simulink model "transmitter_lfsr256_v10"  (R2020a)
settings: Solver=VariableStepAuto  StartTime=0.0  StopTime=3000/128e6  RelTol=1e-3
PreLoadFcn:
  | setup_transmitter
InitFcn:
  | setup_transmitter
values from neighbouring files (symbols this model uses):
  tx_data = fi([1:1:1000], 0, 8, 0)    [setup_transmitter.m]
undefined symbols (not defined in the file or in its folder): a1, a2, a3, a4, a5, a6, a7, a8, AXI4, b1, b2, b3, b4, b5, b6, b7, b8, c1, c2, c3, c4, c5, c6, c7, c8, d1, d2, d3, d4, d5, d6, d7, d8, Lite
libraries: xbsIndex_r4 x30918, dspstat3 x66, hdlsllib x2, simulink x1
(part 1 of 157)
settings Model: Creator=David Northcote  LastModifiedBy=observer
settings SFSimCC: CustomCodeUndefinedFunction=UseInterfaceOnly

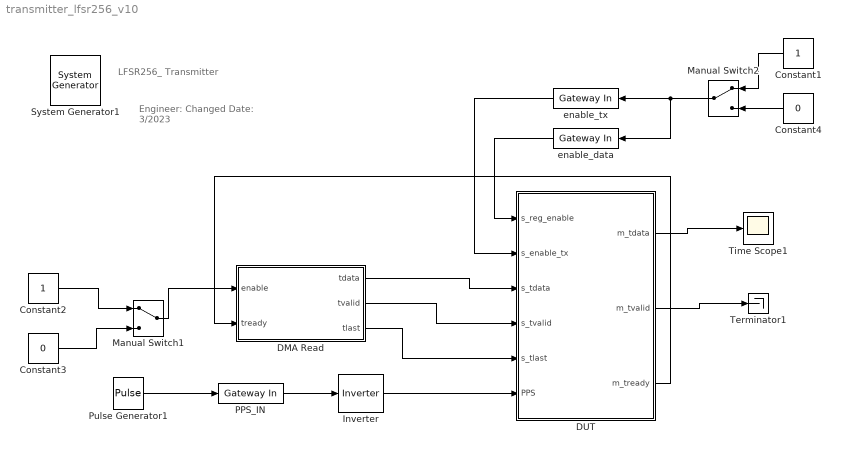
== system transmitter_lfsr256_v10 ==
  note: Engineer:	Changed
      | Date:		3/2023
  note: LFSR256_ Transmitter
  " System Generator1" : xbsIndex_r4/ System Generator  Tag=genX  xilinxfamily=zynquplusRFSOC  part=xczu48dr  speed=-2-e  package=ffvg1517  synthesis_tool=Vivado  clock_wrapper=Clock Enables  directory=/home/observer/Downloads/rfsoc4x2_projects/working_designs/rfsoc_radio_lfsr32x8_983.04MHz_PPS_G/rfsoc_radio/boards/ip/sysgen/transmitter/netlist_lfsr32x8ppsdatabuswidth8_as128  proj_type=Vivado  Synth_file=Vivado Synthesis Defaults  Impl_file=Vivado Implementation Defaults  testbench=off  simulink_period=1/128e6  sysclk_period=(1/128e6)*1e9  dcm_input_clock_period=10  incr_netlist=off  trim_vbits=Everywhere in SubSystem  dbl_ovrd=According to Block Masks  core_generation=According to Block Masks  run_coregen=off  deprecated_control=off  eval_field=0  block_type=sysgen
  Constant1 : Constant  OutDataTypeStr=boolean  SampleTime=1/(128e6)
  Constant2 : Constant  OutDataTypeStr=boolean  SampleTime=1/128e6
  Constant3 : Constant  Value=0  OutDataTypeStr=boolean  SampleTime=1/128e6
  Constant4 : Constant  Value=0  OutDataTypeStr=boolean  SampleTime=1/(128e6)
  DMA Read : Subsystem
  DUT : Subsystem
  Inverter : xbsIndex_r4/Inverter  en=off  latency=0  dbl_ovrd=off  block_type=inv
  Manual Switch1 : ManualSwitch
  Manual Switch2 : ManualSwitch
  PPS_IN : xbsIndex_r4/Gateway In  gui_display_data_type=Boolean  arith_type=Boolean  n_bits=1  bin_pt=0  preci_type=Single  exp_width=8  frac_width=24  rows=1  columns=1  quantization="Round  (unbiased: +/- Inf)"  overflow=Saturate  period=1/128e6  UseAsADC=off  ADCChannel='1'  interface=None  automatic_assignment=on  address_offset=hex2dec('00')  intf_name=''  intf_description=''  default_value=0  timing_constraint=None  locs_specified=off  LOCs={}  io_standard={}  inherit_from_input=off  hdl_port=on  dbl_ovrd=off  block_type=gatewayin
  Pulse Generator1 : DiscretePulseGenerator  PulseType=Time based  Period=1000/128e6  PulseWidth=50
  Terminator1 : Terminator
  Time Scope1 : Scope
  enable_data : xbsIndex_r4/Gateway In  gui_display_data_type=Boolean  arith_type=Boolean  n_bits=16  bin_pt=14  preci_type=Single  exp_width=8  frac_width=24  rows=1  columns=1  quantization="Round  (unbiased: +/- Inf)"  overflow=Saturate  period=1/(128e6)  UseAsADC=off  ADCChannel='1'  interface=AXI4-Lite  automatic_assignment=off  address_offset=0  intf_name=''  intf_description=''  default_value=0  timing_constraint=None  locs_specified=off  LOCs={}  io_standard={}  inherit_from_input=off  hdl_port=on  dbl_ovrd=off  block_type=gatewayin
  enable_tx : xbsIndex_r4/Gateway In  gui_display_data_type=Boolean  arith_type=Boolean  n_bits=16  bin_pt=14  preci_type=Single  exp_width=8  frac_width=24  rows=1  columns=1  quantization="Round  (unbiased: +/- Inf)"  overflow=Saturate  period=1/(128e6)  UseAsADC=off  ADCChannel='1'  interface=AXI4-Lite  automatic_assignment=off  address_offset=4  intf_name=''  intf_description=''  default_value=0  timing_constraint=None  locs_specified=off  LOCs={}  io_standard={}  inherit_from_input=off  hdl_port=on  dbl_ovrd=off  block_type=gatewayin
  wires:
  Constant2/1 -> Manual Switch1/1
  Constant3/1 -> Manual Switch1/2
  Manual Switch1/1 -> DMA Read/1(enable)
  DMA Read/1(tdata) -> DUT/3(s_tdata)
  DMA Read/2(tvalid) -> DUT/4(s_tvalid)
  DMA Read/3(tlast) -> DUT/5(s_tlast)
  DUT/2(m_tvalid) -> Terminator1/1
  enable_data/1 -> DUT/1(s_reg_enable)
  Constant4/1 -> Manual Switch2/2
  Constant1/1 -> Manual Switch2/1
  enable_tx/1 -> DUT/2(s_enable_tx)
  Manual Switch2/1 -> enable_data/1, enable_tx/1
  Pulse Generator1/1 -> PPS_IN/1
  PPS_IN/1 -> Inverter/1
  Inverter/1 -> DUT/6(PPS)
  DUT/1(m_tdata) -> Time Scope1/1
  DUT/3(m_tready) -> DMA Read/2(tready)

== system transmitter_lfsr256_v10/DMA Read ==
  enable : Inport
  tready : Inport  Port=2
  1-D Lookup Table : Lookup_n-D  NumberOfTableDimensions=1  BreakpointsForDimension1=[0:1:numel(tx_data)-1]  Table=tx_data  TableDataTypeStr=Inherit: Inherit from 'Table data'  ExtrapMethod=Clip  InternalRulePriority=Speed  OutDataTypeStr=Inherit: Inherit from 'Table data'  RndMeth=Simplest  InputSameDT=on
  AND : Logic  IconShape=distinctive  AllPortsSameDT=off  OutDataTypeStr=boolean
  AND1 : Logic  IconShape=distinctive  AllPortsSameDT=off  OutDataTypeStr=boolean
  Compare To Constant : simulink/Logic and Bit Operations/Compare To Constant  relop=>=  const=numel(tx_data)-1  OutDataTypeStr=boolean
  Data Type Conversion : DataTypeConversion  OutDataTypeStr=fixdt(0, ceil(log2(numel(tx_data))), 0)
  HDL Counter : hdlsllib/Sources/HDL Counter  CountType=Free running  CountInit=0  CountStep=1  CountMax=25  CountFromType=Initial value  CountFrom=0  CountResetPort=on  CountLoadPort=off  CountEnbPort=on  CountDirPort=off  CountDataType=Unsigned  CountWordLen=32  CountFracLen=0  CountSampTime=1
  Latch : Subsystem
  Rising Edge Detector : Subsystem
  tdata : Outport  VectorParamsAs1DForOutWhenUnconnected=off
  tvalid : Outport  Port=2  VectorParamsAs1DForOutWhenUnconnected=off
  tlast : Outport  Port=3  VectorParamsAs1DForOutWhenUnconnected=off
  wires:
  1-D Lookup Table/1 -> tdata/1
  Compare To Constant/1 -> AND1/1, HDL Counter/1, Latch/2(R)
  enable/1 -> Rising Edge Detector/1(Input)
  HDL Counter/1 -> Compare To Constant/1, Data Type Conversion/1
  Data Type Conversion/1 -> 1-D Lookup Table/1
  Rising Edge Detector/1(Output) -> Latch/1(S)
  AND/1 -> AND1/2, tvalid/1, HDL Counter/2
  Latch/1(Q) -> AND/2
  tready/1 -> AND/1
  AND1/1 -> tlast/1

== system transmitter_lfsr256_v10/DUT ==
  s_reg_enable : Inport
  s_enable_tx : Inport  Port=2
  s_tdata : Inport  Port=3
  s_tvalid : Inport  Port=4
  s_tlast : Inport  Port=5
  PPS : Inport  Port=6
  AXI-Stream Master Interface : Subsystem
  AXI-Stream Slave Interface : Subsystem
  Algorithm : Subsystem
  m_tdata : Outport  VectorParamsAs1DForOutWhenUnconnected=off
  m_tvalid : Outport  Port=2  VectorParamsAs1DForOutWhenUnconnected=off
  m_tready : Outport  Port=3  VectorParamsAs1DForOutWhenUnconnected=off
  wires:
  AXI-Stream Slave Interface/1(m_tdata) -> Algorithm/3(s_tdata)
  AXI-Stream Slave Interface/2(m_tvalid) -> Algorithm/4(s_tvalid)
  s_tvalid/1 -> AXI-Stream Slave Interface/2(s_tvalid)
  s_tdata/1 -> AXI-Stream Slave Interface/1(s_tdata)
  s_tlast/1 -> AXI-Stream Slave Interface/3(s_tlast)
  s_reg_enable/1 -> Algorithm/1(enable_data)
  s_enable_tx/1 -> Algorithm/2(enable_tx)
  Algorithm/1(m_re_tdata) -> AXI-Stream Master Interface/1(s_re_tdata)
  Algorithm/2(m_im_tdata) -> AXI-Stream Master Interface/2(s_im_tdata)
  Algorithm/3(m_tvalid) -> AXI-Stream Master Interface/3(s_tvalid)
  PPS/1 -> Algorithm/5(PPS)
  Algorithm/4(m_tdata) -> AXI-Stream Master Interface/4(s_tdata)
  AXI-Stream Slave Interface/4(m_tlast) -> Algorithm/6(s_tlast)
  Algorithm/5(m_tlast) -> AXI-Stream Master Interface/5(s_tlast)
  AXI-Stream Master Interface/1(m_tdata) -> m_tdata/1
  AXI-Stream Master Interface/2(m_tvalid) -> m_tvalid/1
  AXI-Stream Slave Interface/3(s_tready) -> m_tready/1

== system transmitter_lfsr256_v10/DMA Read/Latch ==
  S : Inport
  R : Inport  Port=2
  Multiport Switch : MultiPortSwitch  DataPortOrder=Zero-based contiguous  Inputs=2  zeroidx=on
  Unit Delay Resettable Synchronous : hdlsllib/Discrete/Unit Delay Resettable Synchronous  InitialCondition=0.0  SampleTime=-1
      settings: SourceProductBaseCode=SL
  Q : Outport  VectorParamsAs1DForOutWhenUnconnected=off
  wires:
  Multiport Switch/1 -> Unit Delay Resettable Synchronous/1, Q/1
  S/1 -> Multiport Switch/3, Multiport Switch/1
  Unit Delay Resettable Synchronous/1 -> Multiport Switch/2
  R/1 -> Unit Delay Resettable Synchronous/2

== system transmitter_lfsr256_v10/DMA Read/Rising Edge Detector ==
  Input : Inport
  AND1 : Logic  IconShape=distinctive  AllPortsSameDT=off  OutDataTypeStr=boolean
  Delay1 : Delay  DelayLength=1
  NOT : Logic  Operator=NOT  IconShape=distinctive  AllPortsSameDT=off  OutDataTypeStr=boolean
  Output : Outport  VectorParamsAs1DForOutWhenUnconnected=off
  wires:
  NOT/1 -> AND1/1
  Input/1 -> AND1/2, Delay1/1
  Delay1/1 -> NOT/1
  AND1/1 -> Output/1

== system transmitter_lfsr256_v10/DUT/AXI-Stream Master Interface ==
  s_re_tdata : Inport
  s_im_tdata : Inport  Port=2
  s_tvalid : Inport  Port=3
  s_tdata : Inport  Port=4
  s_tlast : Inport  Port=5
  AXI FIFO : xbsIndex_r4/AXI FIFO  input_depth_axis=1K  actual_fifo_depth_label=1026  enable_tdata=on  enable_tdest=off  enable_tstrb=off  enable_tready=on  enable_tid=off  enable_tuser=off  enable_tkeep=off  enable_tlast=on  has_aresetn=off  enable_data_counts_axis=off  fifo_implementation_type_axis=Common Clock Block RAM  trim_axipin_name=on  programmable_full_type_axis=Full  full_threshold_assert_value_axis=0  programmable_empty_type_axis=Empty  empty_threshold_assert_value_axis=1022  underflow_flag_axi=0  underflow_sense_axi=Active High  overflow_flag_axi=0  overflow_sense_axi=Active High  simulation_type=fast_sim  block_type=axi_fifo
  Constant : xbsIndex_r4/Constant  const=1.0  gui_display_data_type=Boolean  arith_type=Boolean  n_bits=16  bin_pt=14  preci_type=Single  exp_width=8  frac_width=24  explicit_period=on  period=1/128e6  equ=P=C  opselect=C  inp2=PCIN>>17  opr=+  inp1=P  carry=CIN  dbl_ovrd=off  block_type=constant
  Constant1 : xbsIndex_r4/Constant  const=1.0  gui_display_data_type=Boolean  arith_type=Boolean  n_bits=16  bin_pt=14  preci_type=Single  exp_width=8  frac_width=24  explicit_period=on  period=1/128e6  equ=P=C  opselect=C  inp2=PCIN>>17  opr=+  inp1=P  carry=CIN  dbl_ovrd=off  block_type=constant
  Terminator{1..5} (5 alike) : Terminator
  m_axis_tdata : xbsIndex_r4/Gateway Out  inherit_from_input=on  hdl_port=on  rows=1  columns=1  UseAsDAC=off  DACChannel='1'  interface=None  automatic_assignment=on  address_offset=hex2dec('00')  intf_name=''  intf_description=''  timing_constraint=None  locs_specified=off  LOCs={}  io_standard={}  block_type=gatewayout
  m_axis_tvalid : xbsIndex_r4/Gateway Out  inherit_from_input=on  hdl_port=on  rows=1  columns=1  UseAsDAC=off  DACChannel='1'  interface=None  automatic_assignment=on  address_offset=hex2dec('00')  intf_name=''  intf_description=''  timing_constraint=None  locs_specified=off  LOCs={}  io_standard={}  block_type=gatewayout
  m_tdata : Outport
  m_tvalid : Outport  Port=2  VectorParamsAs1DForOutWhenUnconnected=off
  wires:
  s_im_tdata/1 -> Terminator3/1
  s_re_tdata/1 -> Terminator2/1
  Constant/1 -> AXI FIFO/2
  AXI FIFO/2 -> m_axis_tdata/1
  m_axis_tdata/1 -> m_tdata/1
  AXI FIFO/1 -> m_axis_tvalid/1
  m_axis_tvalid/1 -> m_tvalid/1
  Constant1/1 -> AXI FIFO/1
  AXI FIFO/4 -> Terminator1/1
  s_tdata/1 -> AXI FIFO/3
  s_tlast/1 -> AXI FIFO/4
  AXI FIFO/3 -> Terminator5/1
  s_tvalid/1 -> Terminator4/1

== system transmitter_lfsr256_v10/DUT/AXI-Stream Slave Interface ==
  s_tdata : Inport
  s_tvalid : Inport  Port=2
  s_tlast : Inport  Port=3
  AXI FIFO : xbsIndex_r4/AXI FIFO  input_depth_axis=1K  actual_fifo_depth_label=1026  enable_tdata=on  enable_tdest=off  enable_tstrb=off  enable_tready=on  enable_tid=off  enable_tuser=off  enable_tkeep=off  enable_tlast=on  has_aresetn=off  enable_data_counts_axis=off  fifo_implementation_type_axis=Common Clock Block RAM  trim_axipin_name=on  programmable_full_type_axis=Full  full_threshold_assert_value_axis=0  programmable_empty_type_axis=Empty  empty_threshold_assert_value_axis=1022  underflow_flag_axi=0  underflow_sense_axi=Active High  overflow_flag_axi=0  overflow_sense_axi=Active High  simulation_type=fast_sim  block_type=axi_fifo
  Constant : xbsIndex_r4/Constant  const=1.0  gui_display_data_type=Boolean  arith_type=Boolean  n_bits=16  bin_pt=14  preci_type=Single  exp_width=8  frac_width=24  explicit_period=on  period=1/128e6  equ=P=C  opselect=C  inp2=PCIN>>17  opr=+  inp1=P  carry=CIN  dbl_ovrd=off  block_type=constant
  s_axis_tdata : xbsIndex_r4/Gateway In  gui_display_data_type=Fixed-point  arith_type=Unsigned  n_bits=8  bin_pt=0  preci_type=Single  exp_width=8  frac_width=24  rows=1  columns=1  quantization="Round  (unbiased: +/- Inf)"  overflow=Saturate  period=1/128e6  UseAsADC=off  ADCChannel='1'  interface=None  automatic_assignment=on  address_offset=hex2dec('00')  intf_name=''  intf_description=''  default_value=0  timing_constraint=None  locs_specified=off  LOCs={}  io_standard={}  inherit_from_input=off  hdl_port=on  dbl_ovrd=off  block_type=gatewayin
  s_axis_tlast : xbsIndex_r4/Gateway In  gui_display_data_type=Boolean  arith_type=Boolean  n_bits=1  bin_pt=0  preci_type=Single  exp_width=8  frac_width=24  rows=1  columns=1  quantization="Round  (unbiased: +/- Inf)"  overflow=Saturate  period=1/128e6  UseAsADC=off  ADCChannel='1'  interface=None  automatic_assignment=on  address_offset=hex2dec('00')  intf_name=''  intf_description=''  default_value=0  timing_constraint=None  locs_specified=off  LOCs={}  io_standard={}  inherit_from_input=off  hdl_port=on  dbl_ovrd=off  block_type=gatewayin
  s_axis_tready : xbsIndex_r4/Gateway Out  inherit_from_input=on  hdl_port=on  rows=1  columns=1  UseAsDAC=off  DACChannel='1'  interface=None  automatic_assignment=on  address_offset=hex2dec('00')  intf_name=''  intf_description=''  timing_constraint=None  locs_specified=off  LOCs={}  io_standard={}  block_type=gatewayout
  s_axis_tvalid : xbsIndex_r4/Gateway In  gui_display_data_type=Boolean  arith_type=Boolean  n_bits=16  bin_pt=14  preci_type=Single  exp_width=8  frac_width=24  rows=1  columns=1  quantization="Round  (unbiased: +/- Inf)"  overflow=Saturate  period=1/128e6  UseAsADC=off  ADCChannel='1'  interface=None  automatic_assignment=on  address_offset=hex2dec('00')  intf_name=''  intf_description=''  default_value=0  timing_constraint=None  locs_specified=off  LOCs={}  io_standard={}  inherit_from_input=off  hdl_port=on  dbl_ovrd=off  block_type=gatewayin
  m_tdata : Outport  VectorParamsAs1DForOutWhenUnconnected=off
  m_tvalid : Outport  Port=2  VectorParamsAs1DForOutWhenUnconnected=off
  s_tready : Outport  Port=3  VectorParamsAs1DForOutWhenUnconnected=off
  m_tlast : Outport  Port=4  VectorParamsAs1DForOutWhenUnconnected=off
  wires:
  s_axis_tready/1 -> s_tready/1
  s_tlast/1 -> s_axis_tlast/1
  s_tvalid/1 -> s_axis_tvalid/1
  s_tdata/1 -> s_axis_tdata/1
  s_axis_tvalid/1 -> AXI FIFO/2
  s_axis_tdata/1 -> AXI FIFO/3
  AXI FIFO/2 -> m_tdata/1
  AXI FIFO/1 -> m_tvalid/1
  AXI FIFO/4 -> s_axis_tready/1
  s_axis_tlast/1 -> AXI FIFO/4
  AXI FIFO/3 -> m_tlast/1
  Constant/1 -> AXI FIFO/1

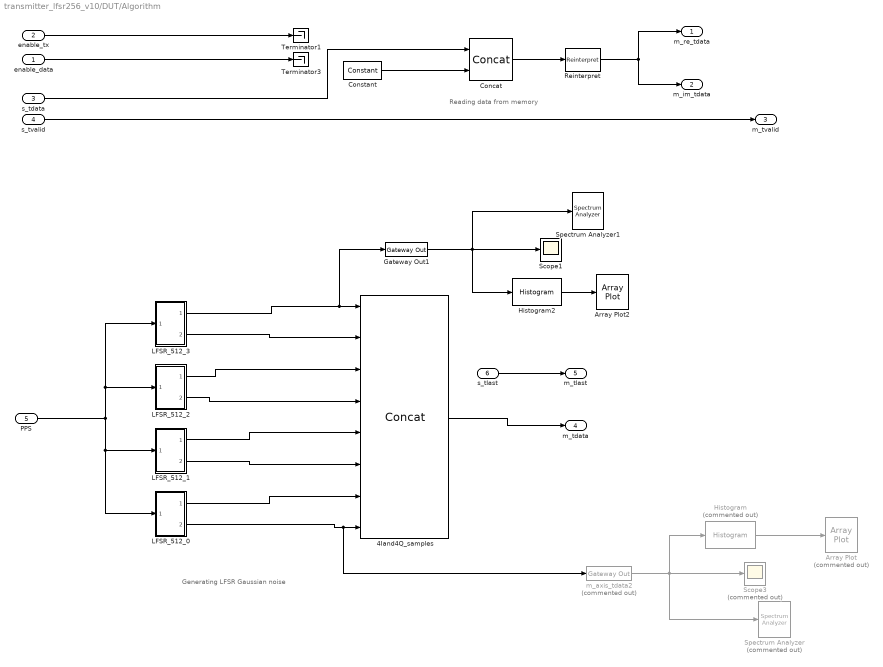
== system transmitter_lfsr256_v10/DUT/Algorithm ==
  note: Generating LFSR Gaussian noise
  note: Reading data from memory
  enable_data : Inport
  enable_tx : Inport  Port=2
  s_tdata : Inport  Port=3
  s_tvalid : Inport  Port=4
  PPS : Inport  Port=5
  s_tlast : Inport  Port=6
  4Iand4Q_samples : xbsIndex_r4/Concat  num_inputs=8  dbl_ovrd=off  block_type=concat
  Array Plot : ArrayPlot  Commented=on
  Array Plot2 : ArrayPlot
  Concat : xbsIndex_r4/Concat  num_inputs=2  dbl_ovrd=off  block_type=concat
  Constant : xbsIndex_r4/Constant  const=0  gui_display_data_type=Fixed-point  arith_type=Unsigned  n_bits=8  bin_pt=0  preci_type=Single  exp_width=8  frac_width=24  explicit_period=on  period=1/128e6  equ=P=C  opselect=C  inp2=PCIN>>17  opr=+  inp1=P  carry=CIN  dbl_ovrd=off  block_type=constant
  Gateway Out1 : xbsIndex_r4/Gateway Out  inherit_from_input=on  hdl_port=off  rows=1  columns=1  UseAsDAC=off  DACChannel='1'  interface=None  automatic_assignment=on  address_offset=hex2dec('00')  intf_name=''  intf_description=''  timing_constraint=None  locs_specified=off  LOCs={}  io_standard={}  block_type=gatewayout
  Histogram : dspstat3/Histogram  Commented=on  umin=-32768  umax=32767  nbins=65536  operateOver=Entire input  Dimension=1  normalized=off  run=on  reset=on  trigtype=None  colComp=on  roundingMode=Floor  overflowMode=off
      settings: SourceProductBaseCode=DS
  Histogram2 : dspstat3/Histogram  umin=-32768  umax=32767  nbins=65536  operateOver=Entire input  Dimension=1  normalized=off  run=on  reset=on  trigtype=None  colComp=on  roundingMode=Floor  overflowMode=off
      settings: SourceProductBaseCode=DS
  LFSR_512_0 : Subsystem
  LFSR_512_1 : Subsystem
  LFSR_512_2 : Subsystem
  LFSR_512_3 : Subsystem
  Reinterpret : xbsIndex_r4/Reinterpret  force_arith_type=off  arith_type=Unsigned  force_bin_pt=on  bin_pt=15  block_type=reinterpret
  Scope1 : Scope
  Scope3 : Scope  Commented=on
  Spectrum Analyzer : SpectrumAnalyzer  Commented=on
  Spectrum Analyzer1 : SpectrumAnalyzer
  Terminator1 : Terminator
  Terminator3 : Terminator
  m_axis_tdata2 : xbsIndex_r4/Gateway Out  Commented=on  inherit_from_input=on  hdl_port=off  rows=1  columns=1  UseAsDAC=off  DACChannel='1'  interface=None  automatic_assignment=on  address_offset=hex2dec('00')  intf_name=''  intf_description=''  timing_constraint=None  locs_specified=off  LOCs={}  io_standard={}  block_type=gatewayout
  m_re_tdata : Outport  VectorParamsAs1DForOutWhenUnconnected=off
  m_im_tdata : Outport  Port=2  VectorParamsAs1DForOutWhenUnconnected=off
  m_tvalid : Outport  Port=3  VectorParamsAs1DForOutWhenUnconnected=off
  m_tdata : Outport  Port=4  VectorParamsAs1DForOutWhenUnconnected=off
  m_tlast : Outport  Port=5  VectorParamsAs1DForOutWhenUnconnected=off
  wires:
  s_tdata/1 -> Concat/1
  enable_data/1 -> Terminator3/1
  s_tvalid/1 -> m_tvalid/1
  enable_tx/1 -> Terminator1/1
  Concat/1 -> Reinterpret/1
  Constant/1 -> Concat/2
  Reinterpret/1 -> m_re_tdata/1, m_im_tdata/1
  4Iand4Q_samples/1 -> m_tdata/1
  m_axis_tdata2/1 -> Histogram/1, Scope3/1, Spectrum Analyzer/1
  Histogram/1 -> Array Plot/1
  Histogram2/1 -> Array Plot2/1
  Gateway Out1/1 -> Histogram2/1, Spectrum Analyzer1/1, Scope1/1
  PPS/1 -> LFSR_512_3/1(PPS), LFSR_512_2/1(PPS), LFSR_512_0/1(PPS), LFSR_512_1/1(PPS)
  s_tlast/1 -> m_tlast/1
  LFSR_512_3/1(Out1) -> Gateway Out1/1, 4Iand4Q_samples/1
  LFSR_512_3/2(Out2) -> 4Iand4Q_samples/2
  LFSR_512_2/1(Out1) -> 4Iand4Q_samples/3
  LFSR_512_2/2(Out2) -> 4Iand4Q_samples/4
  LFSR_512_1/1(Out1) -> 4Iand4Q_samples/5
  LFSR_512_1/2(Out2) -> 4Iand4Q_samples/6
  LFSR_512_0/1(Out1) -> 4Iand4Q_samples/7
  LFSR_512_0/2(Out2) -> m_axis_tdata2/1, 4Iand4Q_samples/8

== system transmitter_lfsr256_v10/DUT/Algorithm/LFSR_512_0 ==
  PPS : Inport
  Concat : xbsIndex_r4/Concat  num_inputs=2  dbl_ovrd=off  block_type=concat
  Concat1 : xbsIndex_r4/Concat  num_inputs=2  dbl_ovrd=off  block_type=concat
  Constant : xbsIndex_r4/Constant  const=0  gui_display_data_type=Fixed-point  arith_type=Unsigned  n_bits=5  bin_pt=0  preci_type=Single  exp_width=8  frac_width=24  explicit_period=on  period=1/128e6  equ=P=C  opselect=C  inp2=PCIN>>17  opr=+  inp1=P  carry=CIN  dbl_ovrd=off  block_type=constant
  Constant1 : xbsIndex_r4/Constant  const=0  gui_display_data_type=Fixed-point  arith_type=Unsigned  n_bits=5  bin_pt=0  preci_type=Single  exp_width=8  frac_width=24  explicit_period=on  period=1/128e6  equ=P=C  opselect=C  inp2=PCIN>>17  opr=+  inp1=P  carry=CIN  dbl_ovrd=off  block_type=constant
  LFSR256_imag : Subsystem
  LFSR256_real : Subsystem
  digital_power_boost : xbsIndex_r4/CMult  const=2  gui_display_data_type=Fixed-point  const_n_bits=2  const_bin_pt=0  float_type=Single  exp_bits=8  fraction_bits=24  en=off  latency=1  precision=User Defined  arith_type=Unsigned  n_bits=16  bin_pt=0  quantization=Truncate  overflow=Saturate  use_behavioral_HDL=off  mem_type=Distributed RAM  pipeline=off  dbl_ovrd=off  block_type=cmult
  digital_power_boost1 : xbsIndex_r4/CMult  const=2  gui_display_data_type=Fixed-point  const_n_bits=2  const_bin_pt=0  float_type=Single  exp_bits=8  fraction_bits=24  en=off  latency=1  precision=User Defined  arith_type=Unsigned  n_bits=16  bin_pt=0  quantization=Truncate  overflow=Saturate  use_behavioral_HDL=off  mem_type=Distributed RAM  pipeline=off  dbl_ovrd=off  block_type=cmult
  offset_comp : xbsIndex_r4/Constant  const=16383  gui_display_data_type=Fixed-point  arith_type=Signed (2's comp)  n_bits=16  bin_pt=0  preci_type=Single  exp_width=8  frac_width=24  explicit_period=on  period=1/128e6  equ=P=C  opselect=C  inp2=PCIN>>17  opr=+  inp1=P  carry=CIN  dbl_ovrd=off  block_type=constant
  offset_comp1 : xbsIndex_r4/Constant  const=16383  gui_display_data_type=Fixed-point  arith_type=Signed (2's comp)  n_bits=16  bin_pt=0  preci_type=Single  exp_width=8  frac_width=24  explicit_period=on  period=1/128e6  equ=P=C  opselect=C  inp2=PCIN>>17  opr=+  inp1=P  carry=CIN  dbl_ovrd=off  block_type=constant
  sfix_imag : xbsIndex_r4/Reinterpret  force_arith_type=on  arith_type="Signed  (2's comp)"  force_bin_pt=on  bin_pt=0  block_type=reinterpret
  sfix_real : xbsIndex_r4/Reinterpret  force_arith_type=on  arith_type="Signed  (2's comp)"  force_bin_pt=on  bin_pt=0  block_type=reinterpret
  shift_to_0th : xbsIndex_r4/AddSub  mode=Subtraction  use_carryin=off  use_carryout=off  en=off  latency=1  precision=User Defined  arith_type="Signed  (2's comp)"  n_bits=16  bin_pt=0  quantization=Truncate  overflow=Wrap  use_behavioral_HDL=off  hw_selection=Fabric  pipelined=off  dbl_ovrd=off  block_type=addsub
  shift_to_0th1 : xbsIndex_r4/AddSub  mode=Subtraction  use_carryin=off  use_carryout=off  en=off  latency=1  precision=User Defined  arith_type="Signed  (2's comp)"  n_bits=16  bin_pt=0  quantization=Truncate  overflow=Wrap  use_behavioral_HDL=off  hw_selection=Fabric  pipelined=off  dbl_ovrd=off  block_type=addsub
  ufix_imag : xbsIndex_r4/Reinterpret  force_arith_type=on  arith_type=Unsigned  force_bin_pt=on  bin_pt=0  block_type=reinterpret
  ufix_real : xbsIndex_r4/Reinterpret  force_arith_type=on  arith_type=Unsigned  force_bin_pt=on  bin_pt=0  block_type=reinterpret
  Out1 : Outport
  Out2 : Outport  Port=2
  wires:
  sfix_real/1 -> shift_to_0th1/1
  offset_comp/1 -> shift_to_0th/2
  shift_to_0th/1 -> ufix_imag/1
  shift_to_0th1/1 -> ufix_real/1
  offset_comp1/1 -> shift_to_0th1/2
  sfix_imag/1 -> shift_to_0th/1
  ufix_imag/1 -> digital_power_boost/1
  digital_power_boost/1 -> Out1/1
  ufix_real/1 -> digital_power_boost1/1
  digital_power_boost1/1 -> Out2/1
  PPS/1 -> LFSR256_real/1(PPS), LFSR256_imag/1(PPS)
  Concat/1 -> sfix_imag/1
  Concat1/1 -> sfix_real/1
  Constant/1 -> Concat/2
  LFSR256_imag/1(Out3) -> Concat/1
  Constant1/1 -> Concat1/2
  LFSR256_real/1(Out4) -> Concat1/1

== system transmitter_lfsr256_v10/DUT/Algorithm/LFSR_512_1 ==
  PPS : Inport
  Concat : xbsIndex_r4/Concat  num_inputs=2  dbl_ovrd=off  block_type=concat
  Concat1 : xbsIndex_r4/Concat  num_inputs=2  dbl_ovrd=off  block_type=concat
  Constant : xbsIndex_r4/Constant  const=0  gui_display_data_type=Fixed-point  arith_type=Unsigned  n_bits=5  bin_pt=0  preci_type=Single  exp_width=8  frac_width=24  explicit_period=on  period=1/128e6  equ=P=C  opselect=C  inp2=PCIN>>17  opr=+  inp1=P  carry=CIN  dbl_ovrd=off  block_type=constant
  Constant1 : xbsIndex_r4/Constant  const=0  gui_display_data_type=Fixed-point  arith_type=Unsigned  n_bits=5  bin_pt=0  preci_type=Single  exp_width=8  frac_width=24  explicit_period=on  period=1/128e6  equ=P=C  opselect=C  inp2=PCIN>>17  opr=+  inp1=P  carry=CIN  dbl_ovrd=off  block_type=constant
  LFSR256_imag : Subsystem
  LFSR256_real : Subsystem
  digital_power_boost : xbsIndex_r4/CMult  const=2  gui_display_data_type=Fixed-point  const_n_bits=2  const_bin_pt=0  float_type=Single  exp_bits=8  fraction_bits=24  en=off  latency=1  precision=User Defined  arith_type=Unsigned  n_bits=16  bin_pt=0  quantization=Truncate  overflow=Saturate  use_behavioral_HDL=off  mem_type=Distributed RAM  pipeline=off  dbl_ovrd=off  block_type=cmult
  digital_power_boost1 : xbsIndex_r4/CMult  const=2  gui_display_data_type=Fixed-point  const_n_bits=2  const_bin_pt=0  float_type=Single  exp_bits=8  fraction_bits=24  en=off  latency=1  precision=User Defined  arith_type=Unsigned  n_bits=16  bin_pt=0  quantization=Truncate  overflow=Saturate  use_behavioral_HDL=off  mem_type=Distributed RAM  pipeline=off  dbl_ovrd=off  block_type=cmult
  offset_comp : xbsIndex_r4/Constant  const=16383  gui_display_data_type=Fixed-point  arith_type=Signed (2's comp)  n_bits=16  bin_pt=0  preci_type=Single  exp_width=8  frac_width=24  explicit_period=on  period=1/128e6  equ=P=C  opselect=C  inp2=PCIN>>17  opr=+  inp1=P  carry=CIN  dbl_ovrd=off  block_type=constant
  offset_comp1 : xbsIndex_r4/Constant  const=16383  gui_display_data_type=Fixed-point  arith_type=Signed (2's comp)  n_bits=16  bin_pt=0  preci_type=Single  exp_width=8  frac_width=24  explicit_period=on  period=1/128e6  equ=P=C  opselect=C  inp2=PCIN>>17  opr=+  inp1=P  carry=CIN  dbl_ovrd=off  block_type=constant
  sfix_imag : xbsIndex_r4/Reinterpret  force_arith_type=on  arith_type="Signed  (2's comp)"  force_bin_pt=on  bin_pt=0  block_type=reinterpret
  sfix_real : xbsIndex_r4/Reinterpret  force_arith_type=on  arith_type="Signed  (2's comp)"  force_bin_pt=on  bin_pt=0  block_type=reinterpret
  shift_to_0th : xbsIndex_r4/AddSub  mode=Subtraction  use_carryin=off  use_carryout=off  en=off  latency=1  precision=User Defined  arith_type="Signed  (2's comp)"  n_bits=16  bin_pt=0  quantization=Truncate  overflow=Wrap  use_behavioral_HDL=off  hw_selection=Fabric  pipelined=off  dbl_ovrd=off  block_type=addsub
  shift_to_0th1 : xbsIndex_r4/AddSub  mode=Subtraction  use_carryin=off  use_carryout=off  en=off  latency=1  precision=User Defined  arith_type="Signed  (2's comp)"  n_bits=16  bin_pt=0  quantization=Truncate  overflow=Wrap  use_behavioral_HDL=off  hw_selection=Fabric  pipelined=off  dbl_ovrd=off  block_type=addsub
  ufix_imag : xbsIndex_r4/Reinterpret  force_arith_type=on  arith_type=Unsigned  force_bin_pt=on  bin_pt=0  block_type=reinterpret
  ufix_real : xbsIndex_r4/Reinterpret  force_arith_type=on  arith_type=Unsigned  force_bin_pt=on  bin_pt=0  block_type=reinterpret
  Out1 : Outport
  Out2 : Outport  Port=2
  wires:
  sfix_real/1 -> shift_to_0th1/1
  offset_comp/1 -> shift_to_0th/2
  shift_to_0th/1 -> ufix_imag/1
  shift_to_0th1/1 -> ufix_real/1
  offset_comp1/1 -> shift_to_0th1/2
  sfix_imag/1 -> shift_to_0th/1
  ufix_imag/1 -> digital_power_boost/1
  digital_power_boost/1 -> Out1/1
  ufix_real/1 -> digital_power_boost1/1
  digital_power_boost1/1 -> Out2/1
  PPS/1 -> LFSR256_real/1(PPS), LFSR256_imag/1(PPS)
  Concat/1 -> sfix_imag/1
  Concat1/1 -> sfix_real/1
  Constant/1 -> Concat/2
  LFSR256_imag/1(Out3) -> Concat/1
  Constant1/1 -> Concat1/2
  LFSR256_real/1(Out4) -> Concat1/1

== system transmitter_lfsr256_v10/DUT/Algorithm/LFSR_512_2 ==
  PPS : Inport
  Concat : xbsIndex_r4/Concat  num_inputs=2  dbl_ovrd=off  block_type=concat
  Concat1 : xbsIndex_r4/Concat  num_inputs=2  dbl_ovrd=off  block_type=concat
  Constant : xbsIndex_r4/Constant  const=0  gui_display_data_type=Fixed-point  arith_type=Unsigned  n_bits=5  bin_pt=0  preci_type=Single  exp_width=8  frac_width=24  explicit_period=on  period=1/128e6  equ=P=C  opselect=C  inp2=PCIN>>17  opr=+  inp1=P  carry=CIN  dbl_ovrd=off  block_type=constant
  Constant1 : xbsIndex_r4/Constant  const=0  gui_display_data_type=Fixed-point  arith_type=Unsigned  n_bits=5  bin_pt=0  preci_type=Single  exp_width=8  frac_width=24  explicit_period=on  period=1/128e6  equ=P=C  opselect=C  inp2=PCIN>>17  opr=+  inp1=P  carry=CIN  dbl_ovrd=off  block_type=constant
  LFSR256_imag : Subsystem
  LFSR256_real : Subsystem
  digital_power_boost : xbsIndex_r4/CMult  const=2  gui_display_data_type=Fixed-point  const_n_bits=2  const_bin_pt=0  float_type=Single  exp_bits=8  fraction_bits=24  en=off  latency=1  precision=User Defined  arith_type=Unsigned  n_bits=16  bin_pt=0  quantization=Truncate  overflow=Saturate  use_behavioral_HDL=off  mem_type=Distributed RAM  pipeline=off  dbl_ovrd=off  block_type=cmult
  digital_power_boost1 : xbsIndex_r4/CMult  const=2  gui_display_data_type=Fixed-point  const_n_bits=2  const_bin_pt=0  float_type=Single  exp_bits=8  fraction_bits=24  en=off  latency=1  precision=User Defined  arith_type=Unsigned  n_bits=16  bin_pt=0  quantization=Truncate  overflow=Saturate  use_behavioral_HDL=off  mem_type=Distributed RAM  pipeline=off  dbl_ovrd=off  block_type=cmult
  offset_comp : xbsIndex_r4/Constant  const=16383  gui_display_data_type=Fixed-point  arith_type=Signed (2's comp)  n_bits=16  bin_pt=0  preci_type=Single  exp_width=8  frac_width=24  explicit_period=on  period=1/128e6  equ=P=C  opselect=C  inp2=PCIN>>17  opr=+  inp1=P  carry=CIN  dbl_ovrd=off  block_type=constant
  offset_comp1 : xbsIndex_r4/Constant  const=16383  gui_display_data_type=Fixed-point  arith_type=Signed (2's comp)  n_bits=16  bin_pt=0  preci_type=Single  exp_width=8  frac_width=24  explicit_period=on  period=1/128e6  equ=P=C  opselect=C  inp2=PCIN>>17  opr=+  inp1=P  carry=CIN  dbl_ovrd=off  block_type=constant
  sfix_imag : xbsIndex_r4/Reinterpret  force_arith_type=on  arith_type="Signed  (2's comp)"  force_bin_pt=on  bin_pt=0  block_type=reinterpret
  sfix_real : xbsIndex_r4/Reinterpret  force_arith_type=on  arith_type="Signed  (2's comp)"  force_bin_pt=on  bin_pt=0  block_type=reinterpret
  shift_to_0th : xbsIndex_r4/AddSub  mode=Subtraction  use_carryin=off  use_carryout=off  en=off  latency=1  precision=User Defined  arith_type="Signed  (2's comp)"  n_bits=16  bin_pt=0  quantization=Truncate  overflow=Wrap  use_behavioral_HDL=off  hw_selection=Fabric  pipelined=off  dbl_ovrd=off  block_type=addsub
  shift_to_0th1 : xbsIndex_r4/AddSub  mode=Subtraction  use_carryin=off  use_carryout=off  en=off  latency=1  precision=User Defined  arith_type="Signed  (2's comp)"  n_bits=16  bin_pt=0  quantization=Truncate  overflow=Wrap  use_behavioral_HDL=off  hw_selection=Fabric  pipelined=off  dbl_ovrd=off  block_type=addsub
  ufix_imag : xbsIndex_r4/Reinterpret  force_arith_type=on  arith_type=Unsigned  force_bin_pt=on  bin_pt=0  block_type=reinterpret
  ufix_real : xbsIndex_r4/Reinterpret  force_arith_type=on  arith_type=Unsigned  force_bin_pt=on  bin_pt=0  block_type=reinterpret
  Out1 : Outport
  Out2 : Outport  Port=2
  wires:
  sfix_real/1 -> shift_to_0th1/1
  offset_comp/1 -> shift_to_0th/2
  shift_to_0th/1 -> ufix_imag/1
  shift_to_0th1/1 -> ufix_real/1
  offset_comp1/1 -> shift_to_0th1/2
  sfix_imag/1 -> shift_to_0th/1
  ufix_imag/1 -> digital_power_boost/1
  digital_power_boost/1 -> Out1/1
  ufix_real/1 -> digital_power_boost1/1
  digital_power_boost1/1 -> Out2/1
  PPS/1 -> LFSR256_real/1(PPS), LFSR256_imag/1(PPS)
  Concat/1 -> sfix_imag/1
  Concat1/1 -> sfix_real/1
  Constant/1 -> Concat/2
  LFSR256_imag/1(Out3) -> Concat/1
  Constant1/1 -> Concat1/2
  LFSR256_real/1(Out4) -> Concat1/1

== system transmitter_lfsr256_v10/DUT/Algorithm/LFSR_512_3 ==
  PPS : Inport
  Concat : xbsIndex_r4/Concat  num_inputs=2  dbl_ovrd=off  block_type=concat
  Concat1 : xbsIndex_r4/Concat  num_inputs=2  dbl_ovrd=off  block_type=concat
  Constant : xbsIndex_r4/Constant  const=0  gui_display_data_type=Fixed-point  arith_type=Unsigned  n_bits=5  bin_pt=0  preci_type=Single  exp_width=8  frac_width=24  explicit_period=on  period=1/128e6  equ=P=C  opselect=C  inp2=PCIN>>17  opr=+  inp1=P  carry=CIN  dbl_ovrd=off  block_type=constant
  Constant1 : xbsIndex_r4/Constant  const=0  gui_display_data_type=Fixed-point  arith_type=Unsigned  n_bits=5  bin_pt=0  preci_type=Single  exp_width=8  frac_width=24  explicit_period=on  period=1/128e6  equ=P=C  opselect=C  inp2=PCIN>>17  opr=+  inp1=P  carry=CIN  dbl_ovrd=off  block_type=constant
  LFSR256_imag : Subsystem
  LFSR256_real : Subsystem
  digital_power_boost : xbsIndex_r4/CMult  const=2  gui_display_data_type=Fixed-point  const_n_bits=2  const_bin_pt=0  float_type=Single  exp_bits=8  fraction_bits=24  en=off  latency=1  precision=User Defined  arith_type=Unsigned  n_bits=16  bin_pt=0  quantization=Truncate  overflow=Saturate  use_behavioral_HDL=off  mem_type=Distributed RAM  pipeline=off  dbl_ovrd=off  block_type=cmult
  digital_power_boost1 : xbsIndex_r4/CMult  const=2  gui_display_data_type=Fixed-point  const_n_bits=2  const_bin_pt=0  float_type=Single  exp_bits=8  fraction_bits=24  en=off  latency=1  precision=User Defined  arith_type=Unsigned  n_bits=16  bin_pt=0  quantization=Truncate  overflow=Saturate  use_behavioral_HDL=off  mem_type=Distributed RAM  pipeline=off  dbl_ovrd=off  block_type=cmult
  offset_comp : xbsIndex_r4/Constant  const=16383  gui_display_data_type=Fixed-point  arith_type=Signed (2's comp)  n_bits=16  bin_pt=0  preci_type=Single  exp_width=8  frac_width=24  explicit_period=on  period=1/128e6  equ=P=C  opselect=C  inp2=PCIN>>17  opr=+  inp1=P  carry=CIN  dbl_ovrd=off  block_type=constant
  offset_comp1 : xbsIndex_r4/Constant  const=16383  gui_display_data_type=Fixed-point  arith_type=Signed (2's comp)  n_bits=16  bin_pt=0  preci_type=Single  exp_width=8  frac_width=24  explicit_period=on  period=1/128e6  equ=P=C  opselect=C  inp2=PCIN>>17  opr=+  inp1=P  carry=CIN  dbl_ovrd=off  block_type=constant
  sfix_imag : xbsIndex_r4/Reinterpret  force_arith_type=on  arith_type="Signed  (2's comp)"  force_bin_pt=on  bin_pt=0  block_type=reinterpret
  sfix_real : xbsIndex_r4/Reinterpret  force_arith_type=on  arith_type="Signed  (2's comp)"  force_bin_pt=on  bin_pt=0  block_type=reinterpret
  shift_to_0th : xbsIndex_r4/AddSub  mode=Subtraction  use_carryin=off  use_carryout=off  en=off  latency=1  precision=User Defined  arith_type="Signed  (2's comp)"  n_bits=16  bin_pt=0  quantization=Truncate  overflow=Wrap  use_behavioral_HDL=off  hw_selection=Fabric  pipelined=off  dbl_ovrd=off  block_type=addsub
  shift_to_0th1 : xbsIndex_r4/AddSub  mode=Subtraction  use_carryin=off  use_carryout=off  en=off  latency=1  precision=User Defined  arith_type="Signed  (2's comp)"  n_bits=16  bin_pt=0  quantization=Truncate  overflow=Wrap  use_behavioral_HDL=off  hw_selection=Fabric  pipelined=off  dbl_ovrd=off  block_type=addsub
  ufix_imag : xbsIndex_r4/Reinterpret  force_arith_type=on  arith_type=Unsigned  force_bin_pt=on  bin_pt=0  block_type=reinterpret
  ufix_real : xbsIndex_r4/Reinterpret  force_arith_type=on  arith_type=Unsigned  force_bin_pt=on  bin_pt=0  block_type=reinterpret
  Out1 : Outport
  Out2 : Outport  Port=2
  wires:
  sfix_real/1 -> shift_to_0th1/1
  offset_comp/1 -> shift_to_0th/2
  shift_to_0th/1 -> ufix_imag/1
  shift_to_0th1/1 -> ufix_real/1
  offset_comp1/1 -> shift_to_0th1/2
  sfix_imag/1 -> shift_to_0th/1
  ufix_imag/1 -> digital_power_boost/1
  digital_power_boost/1 -> Out1/1
  ufix_real/1 -> digital_power_boost1/1
  digital_power_boost1/1 -> Out2/1
  PPS/1 -> LFSR256_real/1(PPS), LFSR256_imag/1(PPS)
  Concat/1 -> sfix_imag/1
  Concat1/1 -> sfix_real/1
  Constant/1 -> Concat/2
  LFSR256_imag/1(Out3) -> Concat/1
  Constant1/1 -> Concat1/2
  LFSR256_real/1(Out4) -> Concat1/1

== system transmitter_lfsr256_v10/DUT/Algorithm/LFSR_512_0/LFSR256_imag ==
  PPS : Inport
  AddSub : xbsIndex_r4/AddSub  mode=Addition  use_carryin=off  use_carryout=off  en=off  latency=as  precision=Full  arith_type=Unsigned  n_bits=16  bin_pt=14  quantization=Truncate  overflow=Wrap  use_behavioral_HDL=off  hw_selection=DSP48  pipelined=on  dbl_ovrd=off  block_type=addsub
  AddSub1 : xbsIndex_r4/AddSub  mode=Addition  use_carryin=off  use_carryout=off  en=off  latency=as  precision=Full  arith_type=Unsigned  n_bits=16  bin_pt=14  quantization=Truncate  overflow=Wrap  use_behavioral_HDL=off  hw_selection=DSP48  pipelined=on  dbl_ovrd=off  block_type=addsub
  AddSub4 : xbsIndex_r4/AddSub  mode=Addition  use_carryin=off  use_carryout=off  en=off  latency=as  precision=Full  arith_type=Unsigned  n_bits=16  bin_pt=14  quantization=Truncate  overflow=Wrap  use_behavioral_HDL=off  hw_selection=DSP48  pipelined=on  dbl_ovrd=off  block_type=addsub
  LFSR64_imag1 : Subsystem  mask{x=a2}
      mask prompts: x "seed1"
  LFSR64_imag2 : Subsystem  mask{x=a3}
      mask prompts: x "seed1"
  LFSR64_imag3 : Subsystem  mask{x=a4}
      mask prompts: x "seed1"
  LFSR64_imag4 : Subsystem  mask{x=a1}
      mask prompts: x "seed1"
  Out3 : Outport
  wires:
  AddSub1/1 -> AddSub4/2
  LFSR64_imag1/1(Out3) -> AddSub/2
  PPS/1 -> LFSR64_imag3/1(PPS), LFSR64_imag2/1(PPS), LFSR64_imag1/1(PPS), LFSR64_imag4/1(PPS)
  AddSub/1 -> AddSub4/1
  LFSR64_imag4/1(Out3) -> AddSub/1
  LFSR64_imag3/1(Out3) -> AddSub1/2
  LFSR64_imag2/1(Out3) -> AddSub1/1
  AddSub4/1 -> Out3/1

== system transmitter_lfsr256_v10/DUT/Algorithm/LFSR_512_0/LFSR256_real ==
  PPS : Inport
  AddSub2 : xbsIndex_r4/AddSub  mode=Addition  use_carryin=off  use_carryout=off  en=off  latency=as  precision=Full  arith_type=Unsigned  n_bits=16  bin_pt=14  quantization=Truncate  overflow=Wrap  use_behavioral_HDL=off  hw_selection=DSP48  pipelined=on  dbl_ovrd=off  block_type=addsub
  AddSub3 : xbsIndex_r4/AddSub  mode=Addition  use_carryin=off  use_carryout=off  en=off  latency=as  precision=Full  arith_type=Unsigned  n_bits=16  bin_pt=14  quantization=Truncate  overflow=Wrap  use_behavioral_HDL=off  hw_selection=DSP48  pipelined=on  dbl_ovrd=off  block_type=addsub
  AddSub5 : xbsIndex_r4/AddSub  mode=Addition  use_carryin=off  use_carryout=off  en=off  latency=as  precision=Full  arith_type=Unsigned  n_bits=16  bin_pt=14  quantization=Truncate  overflow=Wrap  use_behavioral_HDL=off  hw_selection=DSP48  pipelined=on  dbl_ovrd=off  block_type=addsub
  LFSR64_imag1 : Subsystem  mask{x=a6}
      mask prompts: x "seed1"
  LFSR64_imag2 : Subsystem  mask{x=a7}
      mask prompts: x "seed1"
  LFSR64_imag3 : Subsystem  mask{x=a8}
      mask prompts: x "seed1"
  LFSR64_imag4 : Subsystem  mask{x=a5}
      mask prompts: x "seed1"
  Out4 : Outport
  wires:
  LFSR64_imag4/1(Out3) -> AddSub2/1
  LFSR64_imag3/1(Out3) -> AddSub3/2
  AddSub2/1 -> AddSub5/1
  LFSR64_imag1/1(Out3) -> AddSub2/2
  LFSR64_imag2/1(Out3) -> AddSub3/1
  AddSub3/1 -> AddSub5/2
  PPS/1 -> LFSR64_imag3/1(PPS), LFSR64_imag2/1(PPS), LFSR64_imag1/1(PPS), LFSR64_imag4/1(PPS)
  AddSub5/1 -> Out4/1

== system transmitter_lfsr256_v10/DUT/Algorithm/LFSR_512_1/LFSR256_imag ==
  PPS : Inport
  AddSub : xbsIndex_r4/AddSub  mode=Addition  use_carryin=off  use_carryout=off  en=off  latency=as  precision=Full  arith_type=Unsigned  n_bits=16  bin_pt=14  quantization=Truncate  overflow=Wrap  use_behavioral_HDL=off  hw_selection=DSP48  pipelined=on  dbl_ovrd=off  block_type=addsub
  AddSub1 : xbsIndex_r4/AddSub  mode=Addition  use_carryin=off  use_carryout=off  en=off  latency=as  precision=Full  arith_type=Unsigned  n_bits=16  bin_pt=14  quantization=Truncate  overflow=Wrap  use_behavioral_HDL=off  hw_selection=DSP48  pipelined=on  dbl_ovrd=off  block_type=addsub
  AddSub4 : xbsIndex_r4/AddSub  mode=Addition  use_carryin=off  use_carryout=off  en=off  latency=as  precision=Full  arith_type=Unsigned  n_bits=16  bin_pt=14  quantization=Truncate  overflow=Wrap  use_behavioral_HDL=off  hw_selection=DSP48  pipelined=on  dbl_ovrd=off  block_type=addsub
  LFSR64_imag1 : Subsystem  mask{x=b2}
      mask prompts: x "seed1"
  LFSR64_imag2 : Subsystem  mask{x=b3}
      mask prompts: x "seed1"
  LFSR64_imag3 : Subsystem  mask{x=b4}
      mask prompts: x "seed1"
  LFSR64_imag4 : Subsystem  mask{x=b1}
      mask prompts: x "seed1"
  Out3 : Outport
  wires:
  AddSub1/1 -> AddSub4/2
  LFSR64_imag1/1(Out3) -> AddSub/2
  PPS/1 -> LFSR64_imag3/1(PPS), LFSR64_imag2/1(PPS), LFSR64_imag1/1(PPS), LFSR64_imag4/1(PPS)
  AddSub/1 -> AddSub4/1
  LFSR64_imag4/1(Out3) -> AddSub/1
  LFSR64_imag3/1(Out3) -> AddSub1/2
  LFSR64_imag2/1(Out3) -> AddSub1/1
  AddSub4/1 -> Out3/1

== system transmitter_lfsr256_v10/DUT/Algorithm/LFSR_512_1/LFSR256_real ==
  PPS : Inport
  AddSub2 : xbsIndex_r4/AddSub  mode=Addition  use_carryin=off  use_carryout=off  en=off  latency=as  precision=Full  arith_type=Unsigned  n_bits=16  bin_pt=14  quantization=Truncate  overflow=Wrap  use_behavioral_HDL=off  hw_selection=DSP48  pipelined=on  dbl_ovrd=off  block_type=addsub
  AddSub3 : xbsIndex_r4/AddSub  mode=Addition  use_carryin=off  use_carryout=off  en=off  latency=as  precision=Full  arith_type=Unsigned  n_bits=16  bin_pt=14  quantization=Truncate  overflow=Wrap  use_behavioral_HDL=off  hw_selection=DSP48  pipelined=on  dbl_ovrd=off  block_type=addsub
  AddSub5 : xbsIndex_r4/AddSub  mode=Addition  use_carryin=off  use_carryout=off  en=off  latency=as  precision=Full  arith_type=Unsigned  n_bits=16  bin_pt=14  quantization=Truncate  overflow=Wrap  use_behavioral_HDL=off  hw_selection=DSP48  pipelined=on  dbl_ovrd=off  block_type=addsub
  LFSR64_imag1 : Subsystem  mask{x=b6}
      mask prompts: x "seed1"
  LFSR64_imag2 : Subsystem  mask{x=b7}
      mask prompts: x "seed1"
  LFSR64_imag3 : Subsystem  mask{x=b8}
      mask prompts: x "seed1"
  LFSR64_imag4 : Subsystem  mask{x=b5}
      mask prompts: x "seed1"
  Out4 : Outport
  wires:
  LFSR64_imag4/1(Out3) -> AddSub2/1
  LFSR64_imag3/1(Out3) -> AddSub3/2
  AddSub2/1 -> AddSub5/1
  LFSR64_imag1/1(Out3) -> AddSub2/2
  LFSR64_imag2/1(Out3) -> AddSub3/1
  AddSub3/1 -> AddSub5/2
  PPS/1 -> LFSR64_imag3/1(PPS), LFSR64_imag2/1(PPS), LFSR64_imag1/1(PPS), LFSR64_imag4/1(PPS)
  AddSub5/1 -> Out4/1

== system transmitter_lfsr256_v10/DUT/Algorithm/LFSR_512_2/LFSR256_imag ==
  PPS : Inport
  AddSub : xbsIndex_r4/AddSub  mode=Addition  use_carryin=off  use_carryout=off  en=off  latency=as  precision=Full  arith_type=Unsigned  n_bits=16  bin_pt=14  quantization=Truncate  overflow=Wrap  use_behavioral_HDL=off  hw_selection=DSP48  pipelined=on  dbl_ovrd=off  block_type=addsub
  AddSub1 : xbsIndex_r4/AddSub  mode=Addition  use_carryin=off  use_carryout=off  en=off  latency=as  precision=Full  arith_type=Unsigned  n_bits=16  bin_pt=14  quantization=Truncate  overflow=Wrap  use_behavioral_HDL=off  hw_selection=DSP48  pipelined=on  dbl_ovrd=off  block_type=addsub
  AddSub4 : xbsIndex_r4/AddSub  mode=Addition  use_carryin=off  use_carryout=off  en=off  latency=as  precision=Full  arith_type=Unsigned  n_bits=16  bin_pt=14  quantization=Truncate  overflow=Wrap  use_behavioral_HDL=off  hw_selection=DSP48  pipelined=on  dbl_ovrd=off  block_type=addsub
  LFSR64_imag1 : Subsystem  mask{x=c2}
      mask prompts: x "seed1"
  LFSR64_imag2 : Subsystem  mask{x=c3}
      mask prompts: x "seed1"
  LFSR64_imag3 : Subsystem  mask{x=c4}
      mask prompts: x "seed1"
  LFSR64_imag4 : Subsystem  mask{x=c1}
      mask prompts: x "seed1"
  Out3 : Outport
  wires:
  AddSub1/1 -> AddSub4/2
  LFSR64_imag1/1(Out3) -> AddSub/2
  PPS/1 -> LFSR64_imag3/1(PPS), LFSR64_imag2/1(PPS), LFSR64_imag1/1(PPS), LFSR64_imag4/1(PPS)
  AddSub/1 -> AddSub4/1
  LFSR64_imag4/1(Out3) -> AddSub/1
  LFSR64_imag3/1(Out3) -> AddSub1/2
  LFSR64_imag2/1(Out3) -> AddSub1/1
  AddSub4/1 -> Out3/1

== system transmitter_lfsr256_v10/DUT/Algorithm/LFSR_512_2/LFSR256_real ==
  PPS : Inport
  AddSub2 : xbsIndex_r4/AddSub  mode=Addition  use_carryin=off  use_carryout=off  en=off  latency=as  precision=Full  arith_type=Unsigned  n_bits=16  bin_pt=14  quantization=Truncate  overflow=Wrap  use_behavioral_HDL=off  hw_selection=DSP48  pipelined=on  dbl_ovrd=off  block_type=addsub
  AddSub3 : xbsIndex_r4/AddSub  mode=Addition  use_carryin=off  use_carryout=off  en=off  latency=as  precision=Full  arith_type=Unsigned  n_bits=16  bin_pt=14  quantization=Truncate  overflow=Wrap  use_behavioral_HDL=off  hw_selection=DSP48  pipelined=on  dbl_ovrd=off  block_type=addsub
  AddSub5 : xbsIndex_r4/AddSub  mode=Addition  use_carryin=off  use_carryout=off  en=off  latency=as  precision=Full  arith_type=Unsigned  n_bits=16  bin_pt=14  quantization=Truncate  overflow=Wrap  use_behavioral_HDL=off  hw_selection=DSP48  pipelined=on  dbl_ovrd=off  block_type=addsub
  LFSR64_imag1 : Subsystem  mask{x=c6}
      mask prompts: x "seed1"
  LFSR64_imag2 : Subsystem  mask{x=c7}
      mask prompts: x "seed1"
  LFSR64_imag3 : Subsystem  mask{x=c8}
      mask prompts: x "seed1"
  LFSR64_imag4 : Subsystem  mask{x=c5}
      mask prompts: x "seed1"
  Out4 : Outport
  wires:
  LFSR64_imag4/1(Out3) -> AddSub2/1
  LFSR64_imag3/1(Out3) -> AddSub3/2
  AddSub2/1 -> AddSub5/1
  LFSR64_imag1/1(Out3) -> AddSub2/2
  LFSR64_imag2/1(Out3) -> AddSub3/1
  AddSub3/1 -> AddSub5/2
  PPS/1 -> LFSR64_imag3/1(PPS), LFSR64_imag2/1(PPS), LFSR64_imag1/1(PPS), LFSR64_imag4/1(PPS)
  AddSub5/1 -> Out4/1

== system transmitter_lfsr256_v10/DUT/Algorithm/LFSR_512_3/LFSR256_imag ==
  PPS : Inport
  AddSub : xbsIndex_r4/AddSub  mode=Addition  use_carryin=off  use_carryout=off  en=off  latency=as  precision=Full  arith_type=Unsigned  n_bits=16  bin_pt=14  quantization=Truncate  overflow=Wrap  use_behavioral_HDL=off  hw_selection=DSP48  pipelined=on  dbl_ovrd=off  block_type=addsub
  AddSub1 : xbsIndex_r4/AddSub  mode=Addition  use_carryin=off  use_carryout=off  en=off  latency=as  precision=Full  arith_type=Unsigned  n_bits=16  bin_pt=14  quantization=Truncate  overflow=Wrap  use_behavioral_HDL=off  hw_selection=DSP48  pipelined=on  dbl_ovrd=off  block_type=addsub
  AddSub4 : xbsIndex_r4/AddSub  mode=Addition  use_carryin=off  use_carryout=off  en=off  latency=as  precision=Full  arith_type=Unsigned  n_bits=16  bin_pt=14  quantization=Truncate  overflow=Wrap  use_behavioral_HDL=off  hw_selection=DSP48  pipelined=on  dbl_ovrd=off  block_type=addsub
  LFSR64_imag1 : Subsystem  mask{x=d2}
      mask prompts: x "seed1"
  LFSR64_imag2 : Subsystem  mask{x=d3}
      mask prompts: x "seed1"
  LFSR64_imag3 : Subsystem  mask{x=d4}
      mask prompts: x "seed1"
  LFSR64_imag4 : Subsystem  mask{x=d1}
      mask prompts: x "seed1"
  Out3 : Outport
  wires:
  AddSub1/1 -> AddSub4/2
  LFSR64_imag1/1(Out3) -> AddSub/2
  PPS/1 -> LFSR64_imag3/1(PPS), LFSR64_imag2/1(PPS), LFSR64_imag1/1(PPS), LFSR64_imag4/1(PPS)
  AddSub/1 -> AddSub4/1
  LFSR64_imag4/1(Out3) -> AddSub/1
  LFSR64_imag3/1(Out3) -> AddSub1/2
  LFSR64_imag2/1(Out3) -> AddSub1/1
  AddSub4/1 -> Out3/1

== system transmitter_lfsr256_v10/DUT/Algorithm/LFSR_512_3/LFSR256_real ==
  PPS : Inport
  AddSub2 : xbsIndex_r4/AddSub  mode=Addition  use_carryin=off  use_carryout=off  en=off  latency=as  precision=Full  arith_type=Unsigned  n_bits=16  bin_pt=14  quantization=Truncate  overflow=Wrap  use_behavioral_HDL=off  hw_selection=DSP48  pipelined=on  dbl_ovrd=off  block_type=addsub
  AddSub3 : xbsIndex_r4/AddSub  mode=Addition  use_carryin=off  use_carryout=off  en=off  latency=as  precision=Full  arith_type=Unsigned  n_bits=16  bin_pt=14  quantization=Truncate  overflow=Wrap  use_behavioral_HDL=off  hw_selection=DSP48  pipelined=on  dbl_ovrd=off  block_type=addsub
  AddSub5 : xbsIndex_r4/AddSub  mode=Addition  use_carryin=off  use_carryout=off  en=off  latency=as  precision=Full  arith_type=Unsigned  n_bits=16  bin_pt=14  quantization=Truncate  overflow=Wrap  use_behavioral_HDL=off  hw_selection=DSP48  pipelined=on  dbl_ovrd=off  block_type=addsub
  LFSR64_imag1 : Subsystem  mask{x=d6}
      mask prompts: x "seed1"
  LFSR64_imag2 : Subsystem  mask{x=d7}
      mask prompts: x "seed1"
  LFSR64_imag3 : Subsystem  mask{x=d8}
      mask prompts: x "seed1"
  LFSR64_imag4 : Subsystem  mask{x=d5}
      mask prompts: x "seed1"
  Out4 : Outport
  wires:
  LFSR64_imag4/1(Out3) -> AddSub2/1
  LFSR64_imag3/1(Out3) -> AddSub3/2
  AddSub2/1 -> AddSub5/1
  LFSR64_imag1/1(Out3) -> AddSub2/2
  LFSR64_imag2/1(Out3) -> AddSub3/1
  AddSub3/1 -> AddSub5/2
  PPS/1 -> LFSR64_imag3/1(PPS), LFSR64_imag2/1(PPS), LFSR64_imag1/1(PPS), LFSR64_imag4/1(PPS)
  AddSub5/1 -> Out4/1

== system transmitter_lfsr256_v10/DUT/Algorithm/LFSR_512_0/LFSR256_imag/LFSR64_imag1 ==
  PPS : Inport
  32LFSR_0 : Subsystem
  32LFSR_1 : Subsystem
  AddSub : xbsIndex_r4/AddSub  mode=Addition  use_carryin=off  use_carryout=off  en=off  latency=as  precision=Full  arith_type=Unsigned  n_bits=16  bin_pt=14  quantization=Truncate  overflow=Wrap  use_behavioral_HDL=off  hw_selection=DSP48  pipelined=on  dbl_ovrd=off  block_type=addsub
  Slice : xbsIndex_r4/Slice  nbits=8  boolean_output=off  mode=Lower Bit Location + Width  bit1=0  base1=MSB of Input  bit0=0  base0=LSB of Input  dbl_ovrd=off  block_type=slice
  Slice1 : xbsIndex_r4/Slice  nbits=8  boolean_output=off  mode=Lower Bit Location + Width  bit1=0  base1=MSB of Input  bit0=0  base0=LSB of Input  dbl_ovrd=off  block_type=slice
  Out3 : Outport
  wires:
  PPS/1 -> 32LFSR_1/1(In1), 32LFSR_0/1(In1)
  Slice1/1 -> AddSub/2
  AddSub/1 -> Out3/1
  Slice/1 -> AddSub/1
  32LFSR_1/1(Out1) -> Slice1/1
  32LFSR_0/1(Out1) -> Slice/1

== system transmitter_lfsr256_v10/DUT/Algorithm/LFSR_512_0/LFSR256_imag/LFSR64_imag2 ==
  PPS : Inport
  32LFSR_0 : Subsystem
  32LFSR_1 : Subsystem
  AddSub : xbsIndex_r4/AddSub  mode=Addition  use_carryin=off  use_carryout=off  en=off  latency=as  precision=Full  arith_type=Unsigned  n_bits=16  bin_pt=14  quantization=Truncate  overflow=Wrap  use_behavioral_HDL=off  hw_selection=DSP48  pipelined=on  dbl_ovrd=off  block_type=addsub
  Slice : xbsIndex_r4/Slice  nbits=8  boolean_output=off  mode=Lower Bit Location + Width  bit1=0  base1=MSB of Input  bit0=0  base0=LSB of Input  dbl_ovrd=off  block_type=slice
  Slice1 : xbsIndex_r4/Slice  nbits=8  boolean_output=off  mode=Lower Bit Location + Width  bit1=0  base1=MSB of Input  bit0=0  base0=LSB of Input  dbl_ovrd=off  block_type=slice
  Out3 : Outport
  wires:
  PPS/1 -> 32LFSR_1/1(In1), 32LFSR_0/1(In1)
  Slice1/1 -> AddSub/2
  AddSub/1 -> Out3/1
  Slice/1 -> AddSub/1
  32LFSR_1/1(Out1) -> Slice1/1
  32LFSR_0/1(Out1) -> Slice/1
